simulink model "Demo_TrafficFlow_cs"  (Simulink 7.6)
settings: Solver=ode5  SolverMode=SingleTasking  StartTime=0.0  StopTime=60  FixedStep=1/20  RelTol=1e-3
PreLoadFcn:
  | check_NUTS_defined
  | try
  |     evalin('base', 'NUTS_defined;');
  | catch
  |     warningMsg = sprintf('PreScan Warning: \nUsing this model requires that MATLAB is configured for PreScan. The model may not display correctly.\n\n ==> Please open MATLAB with the dedicated buttons provided with PreScan');
  |     disp(warningMsg)
  |     warndlg(warningMsg,'WindowStyle','Modal');
  | end
  | 
  | model_closed = 0;
  | assignin('base',['model_closed_' bdroot],false);
InitFcn:
  | PreScanExperimentInitFcn(bdroot)
StopFcn:
  | PreScanExperimentStopFcn(bdroot)
undefined symbols (not defined in the file or in its folder): Advised_Speed_L1, Advised_Speed_L2, Advised_Speed_L3, DiscIntNeverNeededParam, spare_10, spare_5, spare_6, spare_7, spare_8, spare_9, State_L2, Throughput_L1, Throughput_L2, Throughput_L3, Velocity_L1, Velocity_L2, Velocity_L3
libraries: simulink x58, PreScanSimulinkLibrary x40, simulink_extras x32, PreScanUsefulBlocks x22, simulink_need_slupdate x3
(part 5 of 20)

== system Demo_TrafficFlow_cs/STATE_Nissan_Cabstar_1 ==
  State : Inport  SampleTime=TimeStep
  Acceleration : Subsystem  mask(ToSensorWorld){PortName=Acceleration_Nissan_Cabstar_1, PortIndex=161, PortWidth=3, TimeStep=1/20}
      mask prompts: PortName "Port Name [-]"; PortIndex "Index [-]"; PortWidth "Port Dimensions [-]"; TimeStep "Upload Period [s]"
      mask help: Sends signal(s) from Simulink to the Sensor World
      mask Help:
      | Sends signal(s) from Simulink to the Sensor World.
      | 
      | - Port Name [-]: specifies the name of the output terminal of the Simulink block.
      | - Index [-]: specifies the start position in the output vector sent to the Sensor World
      | - Port dimensions [-]: specifies the width (number of scalars) of the output port
      | - Upload period [s]: specifies the upload period (in seconds) of the port.
      mask Display: port_label('input', 1, PortName)
  Demux : Demux  Outputs=5
  Position : Subsystem  mask(ToSensorWorld){PortName=Position_Nissan_Cabstar_1, PortIndex=155, PortWidth=3, TimeStep=1/20}
      mask prompts: PortName "Port Name [-]"; PortIndex "Index [-]"; PortWidth "Port Dimensions [-]"; TimeStep "Upload Period [s]"
      mask help: Sends signal(s) from Simulink to the Sensor World
      mask Help:
      | Sends signal(s) from Simulink to the Sensor World.
      | 
      | - Port Name [-]: specifies the name of the output terminal of the Simulink block.
      | - Index [-]: specifies the start position in the output vector sent to the Sensor World
      | - Port dimensions [-]: specifies the width (number of scalars) of the output port
      | - Upload period [s]: specifies the upload period (in seconds) of the port.
      mask Display: port_label('input', 1, PortName)
  RPY : Subsystem  mask(ToSensorWorld){PortName=RPY_Nissan_Cabstar_1, PortIndex=164, PortWidth=3, TimeStep=1/20}
      mask prompts: PortName "Port Name [-]"; PortIndex "Index [-]"; PortWidth "Port Dimensions [-]"; TimeStep "Upload Period [s]"
      mask help: Sends signal(s) from Simulink to the Sensor World
      mask Help:
      | Sends signal(s) from Simulink to the Sensor World.
      | 
      | - Port Name [-]: specifies the name of the output terminal of the Simulink block.
      | - Index [-]: specifies the start position in the output vector sent to the Sensor World
      | - Port dimensions [-]: specifies the width (number of scalars) of the output port
      | - Upload period [s]: specifies the upload period (in seconds) of the port.
      mask Display: port_label('input', 1, PortName)
  Velocity : Subsystem  mask(ToSensorWorld){PortName=Velocity_Nissan_Cabstar_1, PortIndex=158, PortWidth=3, TimeStep=1/20}
      mask prompts: PortName "Port Name [-]"; PortIndex "Index [-]"; PortWidth "Port Dimensions [-]"; TimeStep "Upload Period [s]"
      mask help: Sends signal(s) from Simulink to the Sensor World
      mask Help:
      | Sends signal(s) from Simulink to the Sensor World.
      | 
      | - Port Name [-]: specifies the name of the output terminal of the Simulink block.
      | - Index [-]: specifies the start position in the output vector sent to the Sensor World
      | - Port dimensions [-]: specifies the width (number of scalars) of the output port
      | - Upload period [s]: specifies the upload period (in seconds) of the port.
      mask Display: port_label('input', 1, PortName)
  iniBODYAngles wrtVEH [rad] : Constant  Value=[Roll0 Pitch0 0]  SampleTime=TimeStep
  iniVEHAngles wrtGLOB [rad] : Constant  Value=[0 0 Yaw0]  SampleTime=TimeStep
  iniVEHposition wrtGLOB [m] : Constant  Value=[x0 y0 z0]  SampleTime=TimeStep
  state2FullState : PreScanSimulinkLibrary/state2FullState  SystemSampleTime=-1
  velRPY : Subsystem  mask(ToSensorWorld){PortName=velRPY_Nissan_Cabstar_1, PortIndex=167, PortWidth=3, TimeStep=1/20}
      mask prompts: PortName "Port Name [-]"; PortIndex "Index [-]"; PortWidth "Port Dimensions [-]"; TimeStep "Upload Period [s]"
      mask help: Sends signal(s) from Simulink to the Sensor World
      mask Help:
      | Sends signal(s) from Simulink to the Sensor World.
      | 
      | - Port Name [-]: specifies the name of the output terminal of the Simulink block.
      | - Index [-]: specifies the start position in the output vector sent to the Sensor World
      | - Port dimensions [-]: specifies the width (number of scalars) of the output port
      | - Upload period [s]: specifies the upload period (in seconds) of the port.
      mask Display: port_label('input', 1, PortName)
  wires:
  Demux/5 -> velRPY/1(Y)
  Demux/4 -> RPY/1(Y)
  Demux/3 -> Acceleration/1(Y)
  Demux/2 -> Velocity/1(Y)
  Demux/1 -> Position/1(Y)
  State/1 -> state2FullState/1
  state2FullState/1 -> Demux/1
  iniVEHposition wrtGLOB [m]/1 -> state2FullState/2
  iniBODYAngles wrtVEH [rad]/1 -> state2FullState/3
  iniVEHAngles wrtGLOB [rad]/1 -> state2FullState/4

== system Demo_TrafficFlow_cs/STATE_Toyota_Previa_1 ==
  State : Inport  SampleTime=TimeStep
  Acceleration : Subsystem  mask(ToSensorWorld){PortName=Acceleration_Toyota_Previa_1, PortIndex=223, PortWidth=3, TimeStep=1/20}
      mask prompts: PortName "Port Name [-]"; PortIndex "Index [-]"; PortWidth "Port Dimensions [-]"; TimeStep "Upload Period [s]"
      mask help: Sends signal(s) from Simulink to the Sensor World
      mask Help:
      | Sends signal(s) from Simulink to the Sensor World.
      | 
      | - Port Name [-]: specifies the name of the output terminal of the Simulink block.
      | - Index [-]: specifies the start position in the output vector sent to the Sensor World
      | - Port dimensions [-]: specifies the width (number of scalars) of the output port
      | - Upload period [s]: specifies the upload period (in seconds) of the port.
      mask Display: port_label('input', 1, PortName)
  Demux : Demux  Outputs=5
  Position : Subsystem  mask(ToSensorWorld){PortName=Position_Toyota_Previa_1, PortIndex=217, PortWidth=3, TimeStep=1/20}
      mask prompts: PortName "Port Name [-]"; PortIndex "Index [-]"; PortWidth "Port Dimensions [-]"; TimeStep "Upload Period [s]"
      mask help: Sends signal(s) from Simulink to the Sensor World
      mask Help:
      | Sends signal(s) from Simulink to the Sensor World.
      | 
      | - Port Name [-]: specifies the name of the output terminal of the Simulink block.
      | - Index [-]: specifies the start position in the output vector sent to the Sensor World
      | - Port dimensions [-]: specifies the width (number of scalars) of the output port
      | - Upload period [s]: specifies the upload period (in seconds) of the port.
      mask Display: port_label('input', 1, PortName)
  RPY : Subsystem  mask(ToSensorWorld){PortName=RPY_Toyota_Previa_1, PortIndex=226, PortWidth=3, TimeStep=1/20}
      mask prompts: PortName "Port Name [-]"; PortIndex "Index [-]"; PortWidth "Port Dimensions [-]"; TimeStep "Upload Period [s]"
      mask help: Sends signal(s) from Simulink to the Sensor World
      mask Help:
      | Sends signal(s) from Simulink to the Sensor World.
      | 
      | - Port Name [-]: specifies the name of the output terminal of the Simulink block.
      | - Index [-]: specifies the start position in the output vector sent to the Sensor World
      | - Port dimensions [-]: specifies the width (number of scalars) of the output port
      | - Upload period [s]: specifies the upload period (in seconds) of the port.
      mask Display: port_label('input', 1, PortName)
  Velocity : Subsystem  mask(ToSensorWorld){PortName=Velocity_Toyota_Previa_1, PortIndex=220, PortWidth=3, TimeStep=1/20}
      mask prompts: PortName "Port Name [-]"; PortIndex "Index [-]"; PortWidth "Port Dimensions [-]"; TimeStep "Upload Period [s]"
      mask help: Sends signal(s) from Simulink to the Sensor World
      mask Help:
      | Sends signal(s) from Simulink to the Sensor World.
      | 
      | - Port Name [-]: specifies the name of the output terminal of the Simulink block.
      | - Index [-]: specifies the start position in the output vector sent to the Sensor World
      | - Port dimensions [-]: specifies the width (number of scalars) of the output port
      | - Upload period [s]: specifies the upload period (in seconds) of the port.
      mask Display: port_label('input', 1, PortName)
  iniBODYAngles wrtVEH [rad] : Constant  Value=[Roll0 Pitch0 0]  SampleTime=TimeStep
  iniVEHAngles wrtGLOB [rad] : Constant  Value=[0 0 Yaw0]  SampleTime=TimeStep
  iniVEHposition wrtGLOB [m] : Constant  Value=[x0 y0 z0]  SampleTime=TimeStep
  state2FullState : PreScanSimulinkLibrary/state2FullState  SystemSampleTime=-1
  velRPY : Subsystem  mask(ToSensorWorld){PortName=velRPY_Toyota_Previa_1, PortIndex=229, PortWidth=3, TimeStep=1/20}
      mask prompts: PortName "Port Name [-]"; PortIndex "Index [-]"; PortWidth "Port Dimensions [-]"; TimeStep "Upload Period [s]"
      mask help: Sends signal(s) from Simulink to the Sensor World
      mask Help:
      | Sends signal(s) from Simulink to the Sensor World.
      | 
      | - Port Name [-]: specifies the name of the output terminal of the Simulink block.
      | - Index [-]: specifies the start position in the output vector sent to the Sensor World
      | - Port dimensions [-]: specifies the width (number of scalars) of the output port
      | - Upload period [s]: specifies the upload period (in seconds) of the port.
      mask Display: port_label('input', 1, PortName)
  wires:
  Demux/5 -> velRPY/1(Y)
  Demux/4 -> RPY/1(Y)
  Demux/3 -> Acceleration/1(Y)
  Demux/2 -> Velocity/1(Y)
  Demux/1 -> Position/1(Y)
  State/1 -> state2FullState/1
  state2FullState/1 -> Demux/1
  iniVEHposition wrtGLOB [m]/1 -> state2FullState/2
  iniBODYAngles wrtVEH [rad]/1 -> state2FullState/3
  iniVEHAngles wrtGLOB [rad]/1 -> state2FullState/4

== system Demo_TrafficFlow_cs/STATE_Toyota_Yaris_1 ==
  State : Inport  SampleTime=TimeStep
  Acceleration : Subsystem  mask(ToSensorWorld){PortName=Acceleration_Toyota_Yaris_1, PortIndex=192, PortWidth=3, TimeStep=1/20}
      mask prompts: PortName "Port Name [-]"; PortIndex "Index [-]"; PortWidth "Port Dimensions [-]"; TimeStep "Upload Period [s]"
      mask help: Sends signal(s) from Simulink to the Sensor World
      mask Help:
      | Sends signal(s) from Simulink to the Sensor World.
      | 
      | - Port Name [-]: specifies the name of the output terminal of the Simulink block.
      | - Index [-]: specifies the start position in the output vector sent to the Sensor World
      | - Port dimensions [-]: specifies the width (number of scalars) of the output port
      | - Upload period [s]: specifies the upload period (in seconds) of the port.
      mask Display: port_label('input', 1, PortName)
  Demux : Demux  Outputs=5
  Position : Subsystem  mask(ToSensorWorld){PortName=Position_Toyota_Yaris_1, PortIndex=186, PortWidth=3, TimeStep=1/20}
      mask prompts: PortName "Port Name [-]"; PortIndex "Index [-]"; PortWidth "Port Dimensions [-]"; TimeStep "Upload Period [s]"
      mask help: Sends signal(s) from Simulink to the Sensor World
      mask Help:
      | Sends signal(s) from Simulink to the Sensor World.
      | 
      | - Port Name [-]: specifies the name of the output terminal of the Simulink block.
      | - Index [-]: specifies the start position in the output vector sent to the Sensor World
      | - Port dimensions [-]: specifies the width (number of scalars) of the output port
      | - Upload period [s]: specifies the upload period (in seconds) of the port.
      mask Display: port_label('input', 1, PortName)
  RPY : Subsystem  mask(ToSensorWorld){PortName=RPY_Toyota_Yaris_1, PortIndex=195, PortWidth=3, TimeStep=1/20}
      mask prompts: PortName "Port Name [-]"; PortIndex "Index [-]"; PortWidth "Port Dimensions [-]"; TimeStep "Upload Period [s]"
      mask help: Sends signal(s) from Simulink to the Sensor World
      mask Help:
      | Sends signal(s) from Simulink to the Sensor World.
      | 
      | - Port Name [-]: specifies the name of the output terminal of the Simulink block.
      | - Index [-]: specifies the start position in the output vector sent to the Sensor World
      | - Port dimensions [-]: specifies the width (number of scalars) of the output port
      | - Upload period [s]: specifies the upload period (in seconds) of the port.
      mask Display: port_label('input', 1, PortName)
  Velocity : Subsystem  mask(ToSensorWorld){PortName=Velocity_Toyota_Yaris_1, PortIndex=189, PortWidth=3, TimeStep=1/20}
      mask prompts: PortName "Port Name [-]"; PortIndex "Index [-]"; PortWidth "Port Dimensions [-]"; TimeStep "Upload Period [s]"
      mask help: Sends signal(s) from Simulink to the Sensor World
      mask Help:
      | Sends signal(s) from Simulink to the Sensor World.
      | 
      | - Port Name [-]: specifies the name of the output terminal of the Simulink block.
      | - Index [-]: specifies the start position in the output vector sent to the Sensor World
      | - Port dimensions [-]: specifies the width (number of scalars) of the output port
      | - Upload period [s]: specifies the upload period (in seconds) of the port.
      mask Display: port_label('input', 1, PortName)
  iniBODYAngles wrtVEH [rad] : Constant  Value=[Roll0 Pitch0 0]  SampleTime=TimeStep
  iniVEHAngles wrtGLOB [rad] : Constant  Value=[0 0 Yaw0]  SampleTime=TimeStep
  iniVEHposition wrtGLOB [m] : Constant  Value=[x0 y0 z0]  SampleTime=TimeStep
  state2FullState : PreScanSimulinkLibrary/state2FullState  SystemSampleTime=-1
  velRPY : Subsystem  mask(ToSensorWorld){PortName=velRPY_Toyota_Yaris_1, PortIndex=198, PortWidth=3, TimeStep=1/20}
      mask prompts: PortName "Port Name [-]"; PortIndex "Index [-]"; PortWidth "Port Dimensions [-]"; TimeStep "Upload Period [s]"
      mask help: Sends signal(s) from Simulink to the Sensor World
      mask Help:
      | Sends signal(s) from Simulink to the Sensor World.
      | 
      | - Port Name [-]: specifies the name of the output terminal of the Simulink block.
      | - Index [-]: specifies the start position in the output vector sent to the Sensor World
      | - Port dimensions [-]: specifies the width (number of scalars) of the output port
      | - Upload period [s]: specifies the upload period (in seconds) of the port.
      mask Display: port_label('input', 1, PortName)
  wires:
  Demux/5 -> velRPY/1(Y)
  Demux/4 -> RPY/1(Y)
  Demux/3 -> Acceleration/1(Y)
  Demux/2 -> Velocity/1(Y)
  Demux/1 -> Position/1(Y)
  State/1 -> state2FullState/1
  state2FullState/1 -> Demux/1
  iniVEHposition wrtGLOB [m]/1 -> state2FullState/2
  iniBODYAngles wrtVEH [rad]/1 -> state2FullState/3
  iniVEHAngles wrtGLOB [rad]/1 -> state2FullState/4

== system Demo_TrafficFlow_cs/SetActuators ==
  Bus Creator : BusCreator  Inputs=1
  Data Store Read : DataStoreRead  DataStoreName=SimCoreActuators  SampleTime=TimeStep
  SendActuators : M-S-Function  FunctionName=SendActuatorsBlock  Parameters=TimeStep NrOfActuators
  dummy : Outport
  tag scope: SimCoreInfo=global
  wires:
  Bus Creator/1 -> [SimCoreInfo]  "SimCore"
  SendActuators/2 -> Bus Creator/1  "ComputationTime[s]"
  SendActuators/1 -> dummy/1
  Data Store Read/1 -> SendActuators/1

== system Demo_TrafficFlow_cs/SimCoreInfo ==
  Bus Selector : BusSelector  OutputSignals=ComputationTime[s]
  Time : Scope
      settings: TimeRange=10  YMin=0  YMax=0.5  SaveName=ComputationTime
  wires:
  Bus Selector/1 -> Time/1  "<ComputationTime[s]>"
  [SimCoreInfo] -> Bus Selector/1

== system Demo_TrafficFlow_cs/Toyota_Previa_1 ==
  note: Simple Longitudinal Advanced Cruise Control
      | with the following inputs:
      | - Advised  velocity received via Beacon
      | - Range to vehicle in front detected by AIR sensor
  note: Trajectories:
      | - Following path with ACC Velocity
      | - Looping trajectory (Reinsert vehicle on
      |   LANE 3 when triggered by Traffic Controller)
      | - Lane change is randomly executed
      |   when LANE 2 is open for traffic of LANE 3
      |   (misuse of Beacon, as visual interpretation
      |   of matrix sign is not possible)
  SELF_Toyota_Previa_1 : Inport  Tag=SELFInport
  RFOBU_7_Rx : Inport  Tag=OBURxInport  Port=2
  AIR_8 : Inport  Tag=AIRInport  Port=3  OutDataTypeStr=Bus: PRESCAN_AIRSENSORMESSAGE
  AIR_8_Demux : Subsystem  Tag=DemuxAIR  mask(PreScan Demux Block: AIR){DetectionType=BBox, detectableObjects=10}
      mask prompts: DetectionType "Detection Type"; detectableObjects "Max. detectable objects"
      mask help:
      | The Actor Information Receiver sensor provides a fast, simple and generic detection solution which is not tied up to a specific technology and can be used for scenarios in which a simple sensor is needed.
      |
      | For more information, please click Help.
      mask init: same as Demo_TrafficFlow_cs/Audi_A8_1/AIR_1_Demux
      settings: DeleteFcn=PreScanBlockDeleteFcn;
      mask Help: eval('openHelp(411)')
      mask StyleString: popup(Center|BBox|CoG),edit
      mask Display:
      | disp(char('Det. type:         ',['{\it',DetectionTypeStr,'}']),'texmode','on')
      | port_label('output',1,'Range [m]')
      | port_label('output',2,'{\theta} [deg]','texmode','on')
      | port_label('output',3,'{\phi} [deg]','texmode','on')
      | port_label('output',4,'ID [-]','texmode','on')
      | port_label('output',5,'Velocity [m/s]','texmode','on')
      | port_label('output',6,'Heading [deg]','texmode','on')
  Ground_{1..16} (16 alike) : Ground
  Memory : Memory
  Memory1 : Memory
  Memory2 : Memory
  RFOBU_7_Rx_Demux : Subsystem  Tag=DemuxOBURx  mask(PreScan Demux Block:){Technology='RF'}
      mask init:
      | IconTxt = char('','','','Technology:            ',['{\it',Technology,'}']);
      settings: DeleteFcn=PreScanBlockDeleteFcn;
      mask Display:
      | disp(IconTxt,'texmode','on')
      | port_label('output', 1, 'Beacon ID [-]')
      | port_label('output', 2, 'Beacon GPS Lat [deg]')
      | port_label('output', 3, 'Beacon GPS Lon [deg]')
      | port_label('output', 4, 'Beacon GPS Alt [m]')
      | port_label('output', 5, 'BeaconType [-]')
      | port_label('output', 6, 'Advised_Velocity')
      | port_label('output', 7, 'CarID_Lane1')
      | port_label('output', 8, 'CarID_Lane3')
      | port_label('output', 9, 'State_Lane2')
      | port_label('output', 10, 'spare_5')
      | port_label('output', 11, 'spare_6')
      | port_label('output', 12, 'spare_7')
      | port_label('output', 13, 'spare_8')
      | port_label('output', 14, 'spare_9')
      | port_label('output', 15, 'spare_10')
      | port_label('output', 16, 'spare_11')
      | port_label('output', 17, 'spare_12')
      | port_label('output', 18, 'spare_13')
      | port_label('output', 19, 'spare_14')
      | port_label('output', 20, 'spare_15')
  RFOBU_7_Tx_Mux : Subsystem  Tag=MuxOBUTx  mask(PreScan Mux Block:){Technology='RF'}
      mask init:
      | IconTxt = char('','','              Technology:',['{\it                ',Technology,'}']);
      settings: DeleteFcn=PreScanBlockDeleteFcn;
      mask Display:
      | disp(IconTxt,'texmode','on')
      | port_label('input', 1, 'Vehicle Type [-]')
      | port_label('input', 2, 'spare_1')
      | port_label('input', 3, 'spare_2')
      | port_label('input', 4, 'spare_3')
      | port_label('input', 5, 'spare_4')
      | port_label('input', 6, 'spare_5')
      | port_label('input', 7, 'spare_6')
      | port_label('input', 8, 'spare_7')
      | port_label('input', 9, 'spare_8')
      | port_label('input', 10, 'spare_9')
      | port_label('input', 11, 'spare_10')
      | port_label('input', 12, 'spare_11')
      | port_label('input', 13, 'spare_12')
      | port_label('input', 14, 'spare_13')
      | port_label('input', 15, 'spare_14')
      | port_label('input', 16, 'spare_15')
  SELF_Demux : Subsystem  Tag=DemuxSELF
      settings: DeleteFcn=PreScanBlockDeleteFcn;
      mask Type: PreScan Demux Block:
      mask Display:
      | port_label('output',1,'x [m]')
      | port_label('output',2,'y [m]')
      | port_label('output',3,'z [m]','texmode','on')
      | port_label('output',4,'Rotx [deg]','texmode','on')
      | port_label('output',5,'Roty [deg]','texmode','on')
      | port_label('output',6,'Rotz [deg]','texmode','on')
      | port_label('output',7,'GPS lat [deg]','texmode','on')
      | port_label('output',8,'GPS long [deg]','texmode','on')
      | port_label('output',9,'GPS alt [m]','texmode','on')
      | port_label('output',10,'Velocity [m/s]')
      | port_label('output',11,'Heading [deg]')
      | port_label('output',12,'Yaw rate [deg/s]')
  Selector1 : Selector  InputPortWidth=2  IndexOptions=Index vector (dialog)  Indices=[1]  OutputSizes=1
  Selector2 : Selector  InputPortWidth=2  IndexOptions=Index vector (dialog)  Indices=[1]  OutputSizes=1
  Selector3 : Selector  InputPortWidth=2  IndexOptions=Index vector (dialog)  Indices=[1]  OutputSizes=1
  Selector4 : Selector  InputPortWidth=10  IndexOptions=Index vector (dialog)  Indices=[1]  OutputSizes=1
  Selector5 : Selector  InputPortWidth=10  IndexOptions=Index vector (dialog)  Indices=[1]  OutputSizes=1
  Simple_Longitudinal_ACC : Subsystem
  Terminator_{1..33} (33 alike) : Terminator
  Trajectories : Subsystem  Tag=Trajectories
      settings: DeleteFcn=PreScanBlockDeleteFcn;
      mask Display: image(imread('Trajectory.png'));
  STATE_Toyota_Previa_1 : Outport  Tag=StateOutport
  RFOBU_7_Tx : Outport  Tag=AntennaTOutport  Port=2
  wires:
  Selector1/1 -> Memory/1
  Selector2/1 -> Memory2/1
  Selector3/1 -> Memory1/1
  Selector4/1 -> Simple_Longitudinal_ACC/2(Range [m])
  Memory2/1 -> Simple_Longitudinal_ACC/1(Advised Velocity [km/h])
  Selector5/1 -> Simple_Longitudinal_ACC/3(Velocity [m/s])
  Memory/1 -> [state_lane3]
  Memory1/1 -> [state_lane2]
  Simple_Longitudinal_ACC/1(ACC_Velocity [m/s]) -> [ACC_velocity]
  SELF_Toyota_Previa_1/1 -> SELF_Demux/1(SELF)
  RFOBU_7_Rx/1 -> RFOBU_7_Rx_Demux/1(Beacon_Rx)
  AIR_8/1 -> AIR_8_Demux/1(AIR)
  RFOBU_7_Tx_Mux/1(BeaconTx) -> RFOBU_7_Tx/1
  Trajectories/1(State) -> STATE_Toyota_Previa_1/1
  [state_lane2] -> Trajectories/3(StateLANE2 [-])
  [state_lane3] -> Trajectories/2(OBUID_Reinsert [-])
  [ACC_velocity] -> Trajectories/1(Velocity)
  AIR_8_Demux/1(Range [m]) -> Selector4/1
  RFOBU_7_Rx_Demux/6(spare_1) -> Selector2/1
  RFOBU_7_Rx_Demux/8(spare_3) -> Selector1/1
  RFOBU_7_Rx_Demux/9(spare_4) -> Selector3/1
  AIR_8_Demux/5(Velocity [m/s]) -> Selector5/1
  AIR_8_Demux/2(Azimuth [deg]) -> Terminator_1/1
  AIR_8_Demux/3(Elevation [deg]) -> Terminator_2/1
  AIR_8_Demux/4(ID [-]) -> Terminator_3/1
  AIR_8_Demux/6(Heading [deg]) -> Terminator_4/1
  RFOBU_7_Rx_Demux/1(Beacon_ID) -> Terminator_5/1
  RFOBU_7_Rx_Demux/2(GPS_pos_lat_[deg]) -> Terminator_6/1
  RFOBU_7_Rx_Demux/3(GPS_pos_long_[deg]) -> Terminator_7/1
  RFOBU_7_Rx_Demux/4(GPS_pos_alt_[m]) -> Terminator_8/1
  RFOBU_7_Rx_Demux/5(BeaconType [-]) -> Terminator_9/1
  RFOBU_7_Rx_Demux/7(spare_2) -> Terminator_10/1
  RFOBU_7_Rx_Demux/10(spare_5) -> Terminator_11/1
  RFOBU_7_Rx_Demux/11(spare_6) -> Terminator_12/1
  RFOBU_7_Rx_Demux/12(spare_7) -> Terminator_13/1
  RFOBU_7_Rx_Demux/13(spare_8) -> Terminator_14/1
  RFOBU_7_Rx_Demux/14(spare_9) -> Terminator_15/1
  RFOBU_7_Rx_Demux/15(spare_10) -> Terminator_16/1
  RFOBU_7_Rx_Demux/16(spare_11) -> Terminator_17/1
  RFOBU_7_Rx_Demux/17(spare_12) -> Terminator_18/1
  RFOBU_7_Rx_Demux/18(spare_13) -> Terminator_19/1
  RFOBU_7_Rx_Demux/19(spare_14) -> Terminator_20/1
  RFOBU_7_Rx_Demux/20(spare_15) -> Terminator_21/1
  Ground_1/1 -> RFOBU_7_Tx_Mux/1(Vehicle_Type)
  Ground_2/1 -> RFOBU_7_Tx_Mux/2(spare_1)
  Ground_3/1 -> RFOBU_7_Tx_Mux/3(spare_2)
  Ground_4/1 -> RFOBU_7_Tx_Mux/4(spare_3)
  Ground_5/1 -> RFOBU_7_Tx_Mux/5(spare_4)
  Ground_6/1 -> RFOBU_7_Tx_Mux/6(spare_5)
  Ground_7/1 -> RFOBU_7_Tx_Mux/7(spare_6)
  Ground_8/1 -> RFOBU_7_Tx_Mux/8(spare_7)
  Ground_9/1 -> RFOBU_7_Tx_Mux/9(spare_8)
  Ground_10/1 -> RFOBU_7_Tx_Mux/10(spare_9)
  Ground_11/1 -> RFOBU_7_Tx_Mux/11(spare_10)
  Ground_12/1 -> RFOBU_7_Tx_Mux/12(spare_11)
  Ground_13/1 -> RFOBU_7_Tx_Mux/13(spare_12)
  Ground_14/1 -> RFOBU_7_Tx_Mux/14(spare_13)
  Ground_15/1 -> RFOBU_7_Tx_Mux/15(spare_14)
  Ground_16/1 -> RFOBU_7_Tx_Mux/16(spare_15)
  SELF_Demux/1(x [m]) -> Terminator_22/1
  SELF_Demux/2(y [m]) -> Terminator_23/1
  SELF_Demux/3(z [m]) -> Terminator_24/1
  SELF_Demux/4(rotx [deg]) -> Terminator_25/1
  SELF_Demux/5(roty [deg]) -> Terminator_26/1
  SELF_Demux/6(rotz [deg]) -> Terminator_27/1
  SELF_Demux/7(GPS lat [deg]) -> Terminator_28/1
  SELF_Demux/8(GPS long [deg]) -> Terminator_29/1
  SELF_Demux/9(GPS alt [m]) -> Terminator_30/1
  SELF_Demux/10(velocity [m/s]) -> Terminator_31/1
  SELF_Demux/11(heading [deg]) -> Terminator_32/1
  SELF_Demux/12(yaw rate [deg/s]) -> Terminator_33/1

== system Demo_TrafficFlow_cs/Toyota_Yaris_1 ==
  note: Simple Longitudinal Advanced Cruise Control
      | with the following inputs:
      | - Advised  velocity received via Beacon
      | - Range to vehicle in front detected by AIR sensor
  note: Trajectories:
      | - Following path with ACC Velocity
      | - Looping trajectory (Reinsert vehicle on
      |   LANE 3 when triggered by Traffic Controller)
      | - Lane change is randomly executed
      |   when LANE 2 is open for traffic of LANE 3
      |   (misuse of Beacon, as visual interpretation
      |   of matrix sign is not possible)
  SELF_Toyota_Yaris_1 : Inport  Tag=SELFInport
  RFOBU_10_Rx : Inport  Tag=OBURxInport  Port=2
  AIR_7 : Inport  Tag=AIRInport  Port=3  OutDataTypeStr=Bus: PRESCAN_AIRSENSORMESSAGE
  AIR_7_Demux : Subsystem  Tag=DemuxAIR  mask(PreScan Demux Block: AIR){DetectionType=BBox, detectableObjects=10}
      mask prompts: DetectionType "Detection Type"; detectableObjects "Max. detectable objects"
      mask help:
      | The Actor Information Receiver sensor provides a fast, simple and generic detection solution which is not tied up to a specific technology and can be used for scenarios in which a simple sensor is needed.
      |
      | For more information, please click Help.
      mask init: same as Demo_TrafficFlow_cs/Audi_A8_1/AIR_1_Demux
      settings: DeleteFcn=PreScanBlockDeleteFcn;
      mask Help: eval('openHelp(411)')
      mask StyleString: popup(Center|BBox|CoG),edit
      mask Display:
      | disp(char('Det. type:         ',['{\it',DetectionTypeStr,'}']),'texmode','on')
      | port_label('output',1,'Range [m]')
      | port_label('output',2,'{\theta} [deg]','texmode','on')
      | port_label('output',3,'{\phi} [deg]','texmode','on')
      | port_label('output',4,'ID [-]','texmode','on')
      | port_label('output',5,'Velocity [m/s]','texmode','on')
      | port_label('output',6,'Heading [deg]','texmode','on')
  Ground_{1..16} (16 alike) : Ground
  Memory : Memory
  Memory1 : Memory
  Memory2 : Memory
  RFOBU_10_Rx_Demux : Subsystem  Tag=DemuxOBURx  mask(PreScan Demux Block:){Technology='RF'}
      mask init:
      | IconTxt = char('','','','Technology:            ',['{\it',Technology,'}']);
      settings: DeleteFcn=PreScanBlockDeleteFcn;
      mask Display:
      | disp(IconTxt,'texmode','on')
      | port_label('output', 1, 'Beacon ID [-]')
      | port_label('output', 2, 'Beacon GPS Lat [deg]')
      | port_label('output', 3, 'Beacon GPS Lon [deg]')
      | port_label('output', 4, 'Beacon GPS Alt [m]')
      | port_label('output', 5, 'BeaconType [-]')
      | port_label('output', 6, 'Advised_Velocity')
      | port_label('output', 7, 'CarID_Lane1')
      | port_label('output', 8, 'CarID_Lane3')
      | port_label('output', 9, 'State_Lane2')
      | port_label('output', 10, 'spare_5')
      | port_label('output', 11, 'spare_6')
      | port_label('output', 12, 'spare_7')
      | port_label('output', 13, 'spare_8')
      | port_label('output', 14, 'spare_9')
      | port_label('output', 15, 'spare_10')
      | port_label('output', 16, 'spare_11')
      | port_label('output', 17, 'spare_12')
      | port_label('output', 18, 'spare_13')
      | port_label('output', 19, 'spare_14')
      | port_label('output', 20, 'spare_15')
  RFOBU_10_Tx_Mux : Subsystem  Tag=MuxOBUTx  mask(PreScan Mux Block:){Technology='RF'}
      mask init:
      | IconTxt = char('','','              Technology:',['{\it                ',Technology,'}']);
      settings: DeleteFcn=PreScanBlockDeleteFcn;
      mask Display:
      | disp(IconTxt,'texmode','on')
      | port_label('input', 1, 'Vehicle Type [-]')
      | port_label('input', 2, 'spare_1')
      | port_label('input', 3, 'spare_2')
      | port_label('input', 4, 'spare_3')
      | port_label('input', 5, 'spare_4')
      | port_label('input', 6, 'spare_5')
      | port_label('input', 7, 'spare_6')
      | port_label('input', 8, 'spare_7')
      | port_label('input', 9, 'spare_8')
      | port_label('input', 10, 'spare_9')
      | port_label('input', 11, 'spare_10')
      | port_label('input', 12, 'spare_11')
      | port_label('input', 13, 'spare_12')
      | port_label('input', 14, 'spare_13')
      | port_label('input', 15, 'spare_14')
      | port_label('input', 16, 'spare_15')
  SELF_Demux : Subsystem  Tag=DemuxSELF
      settings: DeleteFcn=PreScanBlockDeleteFcn;
      mask Type: PreScan Demux Block:
      mask Display:
      | port_label('output',1,'x [m]')
      | port_label('output',2,'y [m]')
      | port_label('output',3,'z [m]','texmode','on')
      | port_label('output',4,'Rotx [deg]','texmode','on')
      | port_label('output',5,'Roty [deg]','texmode','on')
      | port_label('output',6,'Rotz [deg]','texmode','on')
      | port_label('output',7,'GPS lat [deg]','texmode','on')
      | port_label('output',8,'GPS long [deg]','texmode','on')
      | port_label('output',9,'GPS alt [m]','texmode','on')
      | port_label('output',10,'Velocity [m/s]')
      | port_label('output',11,'Heading [deg]')
      | port_label('output',12,'Yaw rate [deg/s]')
  Selector1 : Selector  InputPortWidth=2  IndexOptions=Index vector (dialog)  Indices=[1]  OutputSizes=1
  Selector2 : Selector  InputPortWidth=2  IndexOptions=Index vector (dialog)  Indices=[1]  OutputSizes=1
  Selector3 : Selector  InputPortWidth=2  IndexOptions=Index vector (dialog)  Indices=[1]  OutputSizes=1
  Selector4 : Selector  InputPortWidth=10  IndexOptions=Index vector (dialog)  Indices=[1]  OutputSizes=1
  Selector5 : Selector  InputPortWidth=10  IndexOptions=Index vector (dialog)  Indices=[1]  OutputSizes=1
  Simple_Longitudinal_ACC : Subsystem
  Terminator_{1..33} (33 alike) : Terminator
  Trajectories : Subsystem  Tag=Trajectories
      settings: DeleteFcn=PreScanBlockDeleteFcn;
      mask Display: image(imread('Trajectory.png'));
  STATE_Toyota_Yaris_1 : Outport  Tag=StateOutport
  RFOBU_10_Tx : Outport  Tag=AntennaTOutport  Port=2
  wires:
  Selector1/1 -> Memory/1
  Selector2/1 -> Memory2/1
  Selector3/1 -> Memory1/1
  Selector4/1 -> Simple_Longitudinal_ACC/2(Range [m])
  Memory2/1 -> Simple_Longitudinal_ACC/1(Advised Velocity [km/h])
  Selector5/1 -> Simple_Longitudinal_ACC/3(Velocity [m/s])
  Memory/1 -> [state_lane3]
  Memory1/1 -> [state_lane2]
  Simple_Longitudinal_ACC/1(ACC_Velocity [m/s]) -> [ACC_velocity]
  SELF_Toyota_Yaris_1/1 -> SELF_Demux/1(SELF)
  RFOBU_10_Rx/1 -> RFOBU_10_Rx_Demux/1(Beacon_Rx)
  AIR_7/1 -> AIR_7_Demux/1(AIR)
  RFOBU_10_Tx_Mux/1(BeaconTx) -> RFOBU_10_Tx/1
  Trajectories/1(State) -> STATE_Toyota_Yaris_1/1
  [state_lane2] -> Trajectories/3(StateLANE2 [-])
  [state_lane3] -> Trajectories/2(OBUID_Reinsert [-])
  [ACC_velocity] -> Trajectories/1(Velocity)
  AIR_7_Demux/1(Range [m]) -> Selector4/1
  RFOBU_10_Rx_Demux/6(spare_1) -> Selector2/1
  RFOBU_10_Rx_Demux/8(spare_3) -> Selector1/1
  RFOBU_10_Rx_Demux/9(spare_4) -> Selector3/1
  AIR_7_Demux/5(Velocity [m/s]) -> Selector5/1
  AIR_7_Demux/2(Azimuth [deg]) -> Terminator_1/1
  AIR_7_Demux/3(Elevation [deg]) -> Terminator_2/1
  AIR_7_Demux/4(ID [-]) -> Terminator_3/1
  AIR_7_Demux/6(Heading [deg]) -> Terminator_4/1
  RFOBU_10_Rx_Demux/1(Beacon_ID) -> Terminator_5/1
  RFOBU_10_Rx_Demux/2(GPS_pos_lat_[deg]) -> Terminator_6/1
  RFOBU_10_Rx_Demux/3(GPS_pos_long_[deg]) -> Terminator_7/1
  RFOBU_10_Rx_Demux/4(GPS_pos_alt_[m]) -> Terminator_8/1
  RFOBU_10_Rx_Demux/5(BeaconType [-]) -> Terminator_9/1
  RFOBU_10_Rx_Demux/7(spare_2) -> Terminator_10/1
  RFOBU_10_Rx_Demux/10(spare_5) -> Terminator_11/1
  RFOBU_10_Rx_Demux/11(spare_6) -> Terminator_12/1
  RFOBU_10_Rx_Demux/12(spare_7) -> Terminator_13/1
  RFOBU_10_Rx_Demux/13(spare_8) -> Terminator_14/1
  RFOBU_10_Rx_Demux/14(spare_9) -> Terminator_15/1
  RFOBU_10_Rx_Demux/15(spare_10) -> Terminator_16/1
  RFOBU_10_Rx_Demux/16(spare_11) -> Terminator_17/1
  RFOBU_10_Rx_Demux/17(spare_12) -> Terminator_18/1
  RFOBU_10_Rx_Demux/18(spare_13) -> Terminator_19/1
  RFOBU_10_Rx_Demux/19(spare_14) -> Terminator_20/1
  RFOBU_10_Rx_Demux/20(spare_15) -> Terminator_21/1
  Ground_1/1 -> RFOBU_10_Tx_Mux/1(Vehicle_Type)
  Ground_2/1 -> RFOBU_10_Tx_Mux/2(spare_1)
  Ground_3/1 -> RFOBU_10_Tx_Mux/3(spare_2)
  Ground_4/1 -> RFOBU_10_Tx_Mux/4(spare_3)
  Ground_5/1 -> RFOBU_10_Tx_Mux/5(spare_4)
  Ground_6/1 -> RFOBU_10_Tx_Mux/6(spare_5)
  Ground_7/1 -> RFOBU_10_Tx_Mux/7(spare_6)
  Ground_8/1 -> RFOBU_10_Tx_Mux/8(spare_7)
  Ground_9/1 -> RFOBU_10_Tx_Mux/9(spare_8)
  Ground_10/1 -> RFOBU_10_Tx_Mux/10(spare_9)
  Ground_11/1 -> RFOBU_10_Tx_Mux/11(spare_10)
  Ground_12/1 -> RFOBU_10_Tx_Mux/12(spare_11)
  Ground_13/1 -> RFOBU_10_Tx_Mux/13(spare_12)
  Ground_14/1 -> RFOBU_10_Tx_Mux/14(spare_13)
  Ground_15/1 -> RFOBU_10_Tx_Mux/15(spare_14)
  Ground_16/1 -> RFOBU_10_Tx_Mux/16(spare_15)
  SELF_Demux/1(x [m]) -> Terminator_22/1
  SELF_Demux/2(y [m]) -> Terminator_23/1
  SELF_Demux/3(z [m]) -> Terminator_24/1
  SELF_Demux/4(rotx [deg]) -> Terminator_25/1
  SELF_Demux/5(roty [deg]) -> Terminator_26/1
  SELF_Demux/6(rotz [deg]) -> Terminator_27/1
  SELF_Demux/7(GPS lat [deg]) -> Terminator_28/1
  SELF_Demux/8(GPS long [deg]) -> Terminator_29/1
  SELF_Demux/9(GPS alt [m]) -> Terminator_30/1
  SELF_Demux/10(velocity [m/s]) -> Terminator_31/1
  SELF_Demux/11(heading [deg]) -> Terminator_32/1
  SELF_Demux/12(yaw rate [deg/s]) -> Terminator_33/1

== system Demo_TrafficFlow_cs/Tripod_1 ==
  note: Loop traffic LANE 3
  RFBeacon_4_Rx : Inport  Tag=BeaconRxInport
  Ground_{1..15} (15 alike) : Ground
  "REINSERT VEHICLE determine ID of Vehicle  which needs to start at beginning of " : Subsystem
  RFBeacon_4_Rx_Demux : Subsystem  Tag=DemuxAntennaR  mask(PreScan Demux Block:){Technology='RF'}
      mask init:
      | IconTxt = char('','','','','Technology:            ',['{\it',Technology,'}']);
      settings: DeleteFcn=PreScanBlockDeleteFcn;
      mask Display:
      | disp(IconTxt,'texmode','on')
      | port_label('output', 1, 'OBU ID [-]')
      | port_label('output', 2, 'OBU Velocity [m/s]')
      | port_label('output', 3, 'OBU Heading [deg]')
      | port_label('output', 4, 'Vehicle Type[-]')
      | port_label('output', 5, 'spare_1')
      | port_label('output', 6, 'spare_2')
      | port_label('output', 7, 'spare_3')
      | port_label('output', 8, 'spare_4')
      | port_label('output', 9, 'spare_5')
      | port_label('output', 10, 'spare_6')
      | port_label('output', 11, 'spare_7')
      | port_label('output', 12, 'spare_8')
      | port_label('output', 13, 'spare_9')
      | port_label('output', 14, 'spare_10')
      | port_label('output', 15, 'spare_11')
      | port_label('output', 16, 'spare_12')
      | port_label('output', 17, 'spare_13')
      | port_label('output', 18, 'spare_14')
      | port_label('output', 19, 'spare_15')
  RFBeacon_4_Tx_Mux : Subsystem  Tag=MuxBeaconTx  mask(PreScan Mux Block:){Technology='RF'}
      mask init:
      | IconTxt = char('','','','              Technology:',['{\it                ',Technology,'}']);
      settings: DeleteFcn=PreScanBlockDeleteFcn;
      mask Display:
      | disp(IconTxt,'texmode','on')
      | port_label('input', 1, 'Beacon Type [-]')
      | port_label('input', 2, 'Advised_Velocity')
      | port_label('input', 3, 'CarID_Lane1')
      | port_label('input', 4, 'CarID_Lane3')
      | port_label('input', 5, 'State_Lane2')
      | port_label('input', 6, 'spare_5')
      | port_label('input', 7, 'spare_6')
      | port_label('input', 8, 'spare_7')
      | port_label('input', 9, 'spare_8')
      | port_label('input', 10, 'spare_9')
      | port_label('input', 11, 'spare_10')
      | port_label('input', 12, 'spare_11')
      | port_label('input', 13, 'spare_12')
      | port_label('input', 14, 'spare_13')
      | port_label('input', 15, 'spare_14')
      | port_label('input', 16, 'spare_15')
  Terminator_{1..18} (18 alike) : Terminator
  RFBeacon_4_Tx : Outport  Tag=AntennaTOutport
  wires:
  [Trigger_Lane3] -> "REINSERT VEHICLE determine ID of Vehicle  which needs to start at beginning of "/1(ReinsertVehicle Trigger (time based))
  RFBeacon_4_Rx/1 -> RFBeacon_4_Rx_Demux/1(Beacon_Rx)
  RFBeacon_4_Tx_Mux/1(BeaconTx) -> RFBeacon_4_Tx/1
  "REINSERT VEHICLE determine ID of Vehicle  which needs to start at beginning of "/1(ID_Reinsert) -> RFBeacon_4_Tx_Mux/4(spare_3)
  RFBeacon_4_Rx_Demux/1(OBU_ID) -> "REINSERT VEHICLE determine ID of Vehicle  which needs to start at beginning of "/2(ID_Vehicle waiting in buffer)
  RFBeacon_4_Rx_Demux/2(Velocity_[m/s]) -> Terminator_1/1
  RFBeacon_4_Rx_Demux/3(Heading [deg]) -> Terminator_2/1
  RFBeacon_4_Rx_Demux/4(vehicle_type) -> Terminator_3/1
  RFBeacon_4_Rx_Demux/5(spare_1) -> Terminator_4/1
  RFBeacon_4_Rx_Demux/6(spare_2) -> Terminator_5/1
  RFBeacon_4_Rx_Demux/7(spare_3) -> Terminator_6/1
  RFBeacon_4_Rx_Demux/8(spare_4) -> Terminator_7/1
  RFBeacon_4_Rx_Demux/9(spare_5) -> Terminator_8/1
  RFBeacon_4_Rx_Demux/10(spare_6) -> Terminator_9/1
  RFBeacon_4_Rx_Demux/11(spare_7) -> Terminator_10/1
  RFBeacon_4_Rx_Demux/12(spare_8) -> Terminator_11/1
  RFBeacon_4_Rx_Demux/13(spare_9) -> Terminator_12/1
  RFBeacon_4_Rx_Demux/14(spare_10) -> Terminator_13/1
  RFBeacon_4_Rx_Demux/15(spare_11) -> Terminator_14/1
  RFBeacon_4_Rx_Demux/16(spare_12) -> Terminator_15/1
  RFBeacon_4_Rx_Demux/17(spare_13) -> Terminator_16/1
  RFBeacon_4_Rx_Demux/18(spare_14) -> Terminator_17/1
  RFBeacon_4_Rx_Demux/19(spare_15) -> Terminator_18/1
  Ground_1/1 -> RFBeacon_4_Tx_Mux/1(BeaconType)
  Ground_2/1 -> RFBeacon_4_Tx_Mux/2(spare_1)
  Ground_3/1 -> RFBeacon_4_Tx_Mux/3(spare_2)
  Ground_4/1 -> RFBeacon_4_Tx_Mux/5(spare_4)
  Ground_5/1 -> RFBeacon_4_Tx_Mux/6(spare_5)
  Ground_6/1 -> RFBeacon_4_Tx_Mux/7(spare_6)
  Ground_7/1 -> RFBeacon_4_Tx_Mux/8(spare_7)
  Ground_8/1 -> RFBeacon_4_Tx_Mux/9(spare_8)
  Ground_9/1 -> RFBeacon_4_Tx_Mux/10(spare_9)
  Ground_10/1 -> RFBeacon_4_Tx_Mux/11(spare_10)
  Ground_11/1 -> RFBeacon_4_Tx_Mux/12(spare_11)
  Ground_12/1 -> RFBeacon_4_Tx_Mux/13(spare_12)
  Ground_13/1 -> RFBeacon_4_Tx_Mux/14(spare_13)
  Ground_14/1 -> RFBeacon_4_Tx_Mux/15(spare_14)
  Ground_15/1 -> RFBeacon_4_Tx_Mux/16(spare_15)

== system Demo_TrafficFlow_cs/Tripod_2 ==
  note: Loop traffic LANE 1
  RFBeacon_5_Rx : Inport  Tag=BeaconRxInport
  Ground_{1..15} (15 alike) : Ground
  "REINSERT VEHICLE determine ID of Vehicle  which needs to start at beginning of " : Subsystem
  RFBeacon_5_Rx_Demux : Subsystem  Tag=DemuxAntennaR  mask(PreScan Demux Block:){Technology='RF'}
      mask init:
      | IconTxt = char('','','','','Technology:            ',['{\it',Technology,'}']);
      settings: DeleteFcn=PreScanBlockDeleteFcn;
      mask Display:
      | disp(IconTxt,'texmode','on')
      | port_label('output', 1, 'OBU ID [-]')
      | port_label('output', 2, 'OBU Velocity [m/s]')
      | port_label('output', 3, 'OBU Heading [deg]')
      | port_label('output', 4, 'Vehicle Type[-]')
      | port_label('output', 5, 'spare_1')
      | port_label('output', 6, 'spare_2')
      | port_label('output', 7, 'spare_3')
      | port_label('output', 8, 'spare_4')
      | port_label('output', 9, 'spare_5')
      | port_label('output', 10, 'spare_6')
      | port_label('output', 11, 'spare_7')
      | port_label('output', 12, 'spare_8')
      | port_label('output', 13, 'spare_9')
      | port_label('output', 14, 'spare_10')
      | port_label('output', 15, 'spare_11')
      | port_label('output', 16, 'spare_12')
      | port_label('output', 17, 'spare_13')
      | port_label('output', 18, 'spare_14')
      | port_label('output', 19, 'spare_15')
  RFBeacon_5_Tx_Mux : Subsystem  Tag=MuxBeaconTx  mask(PreScan Mux Block:){Technology='RF'}
      mask init:
      | IconTxt = char('','','','              Technology:',['{\it                ',Technology,'}']);
      settings: DeleteFcn=PreScanBlockDeleteFcn;
      mask Display:
      | disp(IconTxt,'texmode','on')
      | port_label('input', 1, 'Beacon Type [-]')
      | port_label('input', 2, 'Advised_Velocity')
      | port_label('input', 3, 'CarID_Lane1')
      | port_label('input', 4, 'CarID_Lane3')
      | port_label('input', 5, 'State_Lane2')
      | port_label('input', 6, 'spare_5')
      | port_label('input', 7, 'spare_6')
      | port_label('input', 8, 'spare_7')
      | port_label('input', 9, 'spare_8')
      | port_label('input', 10, 'spare_9')
      | port_label('input', 11, 'spare_10')
      | port_label('input', 12, 'spare_11')
      | port_label('input', 13, 'spare_12')
      | port_label('input', 14, 'spare_13')
      | port_label('input', 15, 'spare_14')
      | port_label('input', 16, 'spare_15')
  Terminator_{1..18} (18 alike) : Terminator
  RFBeacon_5_Tx : Outport  Tag=AntennaTOutport
  wires:
  [Trigger_Lane1] -> "REINSERT VEHICLE determine ID of Vehicle  which needs to start at beginning of "/1(ReinsertVehicle Trigger (time based))
  RFBeacon_5_Rx/1 -> RFBeacon_5_Rx_Demux/1(Beacon_Rx)
  RFBeacon_5_Tx_Mux/1(BeaconTx) -> RFBeacon_5_Tx/1
  "REINSERT VEHICLE determine ID of Vehicle  which needs to start at beginning of "/1(ID_Reinsert) -> RFBeacon_5_Tx_Mux/3(spare_2)
  RFBeacon_5_Rx_Demux/1(OBU_ID) -> "REINSERT VEHICLE determine ID of Vehicle  which needs to start at beginning of "/2(ID_Vehicle waiting in buffer)
  RFBeacon_5_Rx_Demux/2(Velocity_[m/s]) -> Terminator_1/1
  RFBeacon_5_Rx_Demux/3(Heading [deg]) -> Terminator_2/1
  RFBeacon_5_Rx_Demux/4(vehicle_type) -> Terminator_3/1
  RFBeacon_5_Rx_Demux/5(spare_1) -> Terminator_4/1
  RFBeacon_5_Rx_Demux/6(spare_2) -> Terminator_5/1
  RFBeacon_5_Rx_Demux/7(spare_3) -> Terminator_6/1
  RFBeacon_5_Rx_Demux/8(spare_4) -> Terminator_7/1
  RFBeacon_5_Rx_Demux/9(spare_5) -> Terminator_8/1
  RFBeacon_5_Rx_Demux/10(spare_6) -> Terminator_9/1
  RFBeacon_5_Rx_Demux/11(spare_7) -> Terminator_10/1
  RFBeacon_5_Rx_Demux/12(spare_8) -> Terminator_11/1
  RFBeacon_5_Rx_Demux/13(spare_9) -> Terminator_12/1
  RFBeacon_5_Rx_Demux/14(spare_10) -> Terminator_13/1
  RFBeacon_5_Rx_Demux/15(spare_11) -> Terminator_14/1
  RFBeacon_5_Rx_Demux/16(spare_12) -> Terminator_15/1
  RFBeacon_5_Rx_Demux/17(spare_13) -> Terminator_16/1
  RFBeacon_5_Rx_Demux/18(spare_14) -> Terminator_17/1
  RFBeacon_5_Rx_Demux/19(spare_15) -> Terminator_18/1
  Ground_1/1 -> RFBeacon_5_Tx_Mux/1(BeaconType)
  Ground_2/1 -> RFBeacon_5_Tx_Mux/2(spare_1)
  Ground_3/1 -> RFBeacon_5_Tx_Mux/4(spare_3)
  Ground_4/1 -> RFBeacon_5_Tx_Mux/5(spare_4)
  Ground_5/1 -> RFBeacon_5_Tx_Mux/6(spare_5)
  Ground_6/1 -> RFBeacon_5_Tx_Mux/7(spare_6)
  Ground_7/1 -> RFBeacon_5_Tx_Mux/8(spare_7)
  Ground_8/1 -> RFBeacon_5_Tx_Mux/9(spare_8)
  Ground_9/1 -> RFBeacon_5_Tx_Mux/10(spare_9)
  Ground_10/1 -> RFBeacon_5_Tx_Mux/11(spare_10)
  Ground_11/1 -> RFBeacon_5_Tx_Mux/12(spare_11)
  Ground_12/1 -> RFBeacon_5_Tx_Mux/13(spare_12)
  Ground_13/1 -> RFBeacon_5_Tx_Mux/14(spare_13)
  Ground_14/1 -> RFBeacon_5_Tx_Mux/15(spare_14)
  Ground_15/1 -> RFBeacon_5_Tx_Mux/16(spare_15)
